simulink model "AmbTFilMgr"  (R2022b)
settings: Solver=FixedStepDiscrete  StartTime=0.0  StopTime=10.0  FixedStep=1  RelTol=1e-3
settings Model: Creator=blue.ge  LastModifiedBy=blue.ge
settings DataIOCC: SaveFormat=StructureWithTime
settings OptimizationCC: DefaultParameterBehavior=Inlined  EnhancedBackFolding=on  PassReuseOutputArgsAs=Individual arguments  ZeroExternalMemoryAtStartup=off  ZeroInternalMemoryAtStartup=off  OptimizeBlockOrder=speed  DifferentSizesBufferReuse=on  OptimizationCustomize=off  EfficientTunableParamExpr=on
settings DebuggingCC: ParameterTunabilityLossMsg=error
settings ModelReferenceCC: ModelReferenceNumInstancesAllowed=Single
settings SFSimCC: MATLABDynamicMemAlloc=off
settings RTWCC: Description=AUTOSAR  SystemTargetFile=autosar.tlc  GenCodeOnly=on  TemplateMakefile=ert_default_tmf
settings CodeAppCC: ForceParamTrailComments=on  IgnoreCustomStorageClasses=off  OperatorAnnotations=on  SimulinkDataObjDesc=on  SFDataObjDesc=on  InsertBlockDesc=on
settings STFCustomTargetCC: TargetLangStandard=C99 (ISO)  MultiwordLength=256  ParMdlRefBuildCompliant=on  CompOptLevelCompliant=on  IncludeMdlTerminateFcn=off  CombineOutputUpdateFcns=on  SuppressErrorStatus=on  ERTCustomFileBanners=on  SupportNonFinite=off  SupportComplex=off  SupportContinuousTime=off  SupportNonInlinedSFcns=off  RemoveResetFunc=on  MATLABClassNameForMDSCustomization=Simulink.SoftwareTarget.ERTCustomization  AutosarCompliant=on  UseToolchainInfoCompliant=on  LookupTableObjectStructAxisOrder=2,1,3,4,...  LUTObjectStructOrderEvenSpacing=Size,Table,Breakpoints  SystemTargetFile=autosar.tlc
settings ERTTargetCC: TargetLangStandard=C99 (ISO)  ConcurrentExecutionCompliant=off  IncludeMdlTerminateFcn=off  SuppressErrorStatus=on  SupportNonFinite=off  SupportComplex=off  ModelStepFunctionPrototypeControlCompliant=off  CPPClassGenCompliant=off  AutosarCompliant=on  LookupTableObjectStructAxisOrder=2,1,3,4,...  LUTObjectStructOrderEvenSpacing=Size,Table,Breakpoints  InlinedParameterPlacement=NonHierarchical  ERTCustomFileTemplate=example_file_process.tlc

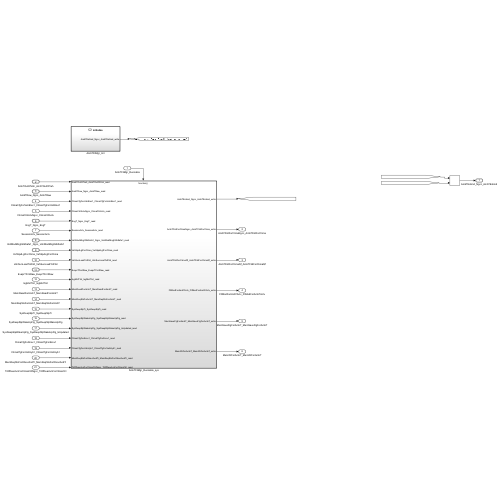
[thumbnail: Simulink's stored preview of the top-level diagram]
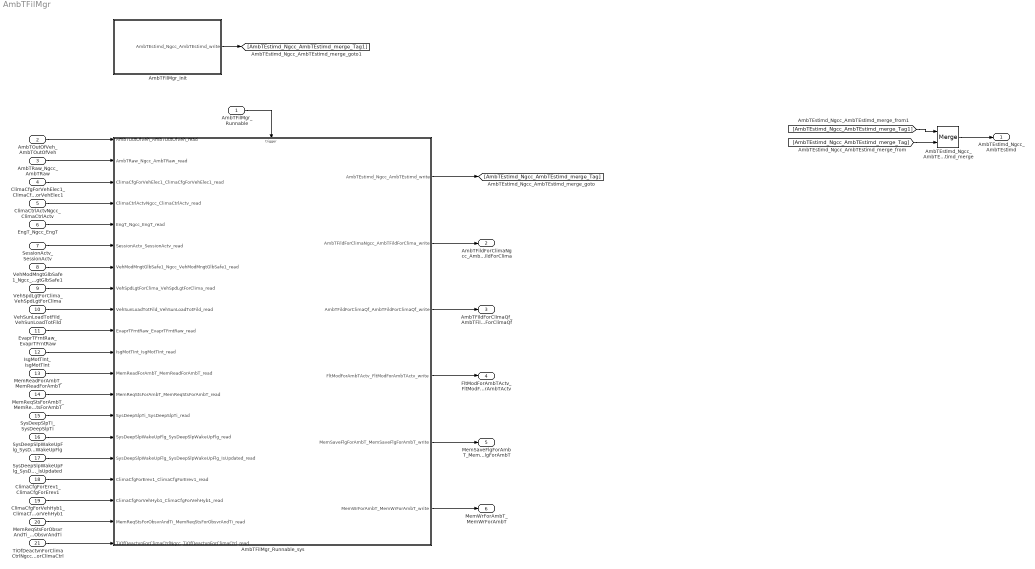
== system AmbTFilMgr ==
  AmbTFilMgr_Runnable : Inport  SampleTime=1  OutputFunctionCall=on
  AmbTOutOfVeh_AmbTOutOfVeh : Inport  Port=2  OutDataTypeStr=Bus: AmbTOutOfVeh  PortDimensions=1  BusOutputAsStruct=on
  AmbTRaw_Ngcc_AmbTRaw : Inport  Port=3  OutDataTypeStr=Bus: AmbTWithQly  PortDimensions=1  BusOutputAsStruct=on
  ClimaCfgForVehElec1_ClimaCfgForVehElec1 : Inport  Port=4  OutDataTypeStr=Boolean  PortDimensions=1
  ClimaCtrlActvNgcc_ClimaCtrlActv : Inport  Port=5  OutDataTypeStr=Boolean  PortDimensions=1
  EngT_Ngcc_EngT : Inport  Port=6  OutDataTypeStr=Bus: EngT  PortDimensions=1  BusOutputAsStruct=on
  SessionActv_SessionActv : Inport  Port=7  OutDataTypeStr=Enum: NoYes1  PortDimensions=1
  VehModMngtGlbSafe1_Ngcc_VehModMngtGlbSafe1 : Inport  Port=8  OutDataTypeStr=Bus: VehModMngtGlbSafe1  PortDimensions=1  BusOutputAsStruct=on
  VehSpdLgtForClima_VehSpdLgtForClima : Inport  Port=9  OutDataTypeStr=Bus: VehSpdLgtForClima  PortDimensions=1  BusOutputAsStruct=on
  VehSunLoadTotFild_VehSunLoadTotFild : Inport  Port=10  OutDataTypeStr=SunLoad30  PortDimensions=1
  EvaprTFrntRaw_EvaprTFrntRaw : Inport  Port=11  OutDataTypeStr=Bus: EvaprTFrnt  PortDimensions=1  BusOutputAsStruct=on
  IsgMotTInt_IsgMotTInt : Inport  Port=12  OutDataTypeStr=T3  PortDimensions=1
  MemReadForAmbT_MemReadForAmbT : Inport  Port=13  OutDataTypeStr=T30  PortDimensions=1
  MemReqStsForAmbT_MemReqStsForAmbT : Inport  Port=14  OutDataTypeStr=Enum: MemReqSts  PortDimensions=1
  SysDeepSlpTi_SysDeepSlpTi : Inport  Port=15  OutDataTypeStr=CarTiGlb  PortDimensions=1
  SysDeepSlpWakeUpFlg_SysDeepSlpWakeUpFlg : Inport  Port=16  OutDataTypeStr=Boolean  PortDimensions=1
  SysDeepSlpWakeUpFlg_SysDeepSlpWakeUpFlg_IsUpdated : Inport  Port=17  OutDataTypeStr=boolean  PortDimensions=1
  ClimaCfgForErev1_ClimaCfgForErev1 : Inport  Port=18  OutDataTypeStr=Boolean  PortDimensions=1
  ClimaCfgForVehHyb1_ClimaCfgForVehHyb1 : Inport  Port=19  OutDataTypeStr=Boolean  PortDimensions=1
  MemReqStsForObsvrAndTi_MemReqStsForObsvrAndTi : Inport  Port=20  OutDataTypeStr=Enum: MemReqSts  PortDimensions=1
  TiOfDeactvnForClimaCtrlNgcc_TiOfDeactvnForClimaCtrl : Inport  Port=21  OutDataTypeStr=Ti33  PortDimensions=1
  AmbTEstimd_Ngcc_AmbTEstimd_merge : Merge
  AmbTFilMgr_Init : Subsystem
  AmbTFilMgr_Runnable_sys : Subsystem
  AmbTEstimd_Ngcc_AmbTEstimd : Outport  OutDataTypeStr=Bus: AmbTEstimd  PortDimensions=1  BusOutputAsStruct=on
  AmbTFildForClimaNgcc_AmbTFildForClima : Outport  Port=2  OutDataTypeStr=T30  PortDimensions=1
  AmbTFildForClimaQf_AmbTFildForClimaQf : Outport  Port=3  OutDataTypeStr=Enum: GenQf1  PortDimensions=1
  FltModForAmbTActv_FltModForAmbTActv : Outport  Port=4  OutDataTypeStr=Boolean  PortDimensions=1
  MemSaveFlgForAmbT_MemSaveFlgForAmbT : Outport  Port=5  OutDataTypeStr=Boolean  PortDimensions=1
  MemWrForAmbT_MemWrForAmbT : Outport  Port=6  OutDataTypeStr=T30  PortDimensions=1
  wires:
  AmbTOutOfVeh_AmbTOutOfVeh/1 -> AmbTFilMgr_Runnable_sys/1(AmbTOutOfVeh_AmbTOutOfVeh_read)
  AmbTRaw_Ngcc_AmbTRaw/1 -> AmbTFilMgr_Runnable_sys/2(AmbTRaw_Ngcc_AmbTRaw_read)
  ClimaCfgForVehElec1_ClimaCfgForVehElec1/1 -> AmbTFilMgr_Runnable_sys/3(ClimaCfgForVehElec1_ClimaCfgForVehElec1_read)
  ClimaCtrlActvNgcc_ClimaCtrlActv/1 -> AmbTFilMgr_Runnable_sys/4(ClimaCtrlActvNgcc_ClimaCtrlActv_read)
  EngT_Ngcc_EngT/1 -> AmbTFilMgr_Runnable_sys/5(EngT_Ngcc_EngT_read)
  SessionActv_SessionActv/1 -> AmbTFilMgr_Runnable_sys/6(SessionActv_SessionActv_read)
  VehModMngtGlbSafe1_Ngcc_VehModMngtGlbSafe1/1 -> AmbTFilMgr_Runnable_sys/7(VehModMngtGlbSafe1_Ngcc_VehModMngtGlbSafe1_read)
  VehSpdLgtForClima_VehSpdLgtForClima/1 -> AmbTFilMgr_Runnable_sys/8(VehSpdLgtForClima_VehSpdLgtForClima_read)
  VehSunLoadTotFild_VehSunLoadTotFild/1 -> AmbTFilMgr_Runnable_sys/9(VehSunLoadTotFild_VehSunLoadTotFild_read)
  EvaprTFrntRaw_EvaprTFrntRaw/1 -> AmbTFilMgr_Runnable_sys/10(EvaprTFrntRaw_EvaprTFrntRaw_read)
  IsgMotTInt_IsgMotTInt/1 -> AmbTFilMgr_Runnable_sys/11(IsgMotTInt_IsgMotTInt_read)
  MemReadForAmbT_MemReadForAmbT/1 -> AmbTFilMgr_Runnable_sys/12(MemReadForAmbT_MemReadForAmbT_read)
  MemReqStsForAmbT_MemReqStsForAmbT/1 -> AmbTFilMgr_Runnable_sys/13(MemReqStsForAmbT_MemReqStsForAmbT_read)
  SysDeepSlpTi_SysDeepSlpTi/1 -> AmbTFilMgr_Runnable_sys/14(SysDeepSlpTi_SysDeepSlpTi_read)
  SysDeepSlpWakeUpFlg_SysDeepSlpWakeUpFlg/1 -> AmbTFilMgr_Runnable_sys/15(SysDeepSlpWakeUpFlg_SysDeepSlpWakeUpFlg_read)
  SysDeepSlpWakeUpFlg_SysDeepSlpWakeUpFlg_IsUpdated/1 -> AmbTFilMgr_Runnable_sys/16(SysDeepSlpWakeUpFlg_SysDeepSlpWakeUpFlg_IsUpdated_read)
  ClimaCfgForErev1_ClimaCfgForErev1/1 -> AmbTFilMgr_Runnable_sys/17(ClimaCfgForErev1_ClimaCfgForErev1_read)
  ClimaCfgForVehHyb1_ClimaCfgForVehHyb1/1 -> AmbTFilMgr_Runnable_sys/18(ClimaCfgForVehHyb1_ClimaCfgForVehHyb1_read)
  MemReqStsForObsvrAndTi_MemReqStsForObsvrAndTi/1 -> AmbTFilMgr_Runnable_sys/19(MemReqStsForObsvrAndTi_MemReqStsForObsvrAndTi_read)
  TiOfDeactvnForClimaCtrlNgcc_TiOfDeactvnForClimaCtrl/1 -> AmbTFilMgr_Runnable_sys/20(TiOfDeactvnForClimaCtrlNgcc_TiOfDeactvnForClimaCtrl_read)
  AmbTEstimd_Ngcc_AmbTEstimd_merge/1 -> AmbTEstimd_Ngcc_AmbTEstimd/1
  AmbTFilMgr_Runnable_sys/2(AmbTFildForClimaNgcc_AmbTFildForClima_write) -> AmbTFildForClimaNgcc_AmbTFildForClima/1
  AmbTFilMgr_Runnable_sys/3(AmbTFildForClimaQf_AmbTFildForClimaQf_write) -> AmbTFildForClimaQf_AmbTFildForClimaQf/1
  AmbTFilMgr_Runnable_sys/4(FltModForAmbTActv_FltModForAmbTActv_write) -> FltModForAmbTActv_FltModForAmbTActv/1
  AmbTFilMgr_Runnable_sys/5(MemSaveFlgForAmbT_MemSaveFlgForAmbT_write) -> MemSaveFlgForAmbT_MemSaveFlgForAmbT/1
  AmbTFilMgr_Runnable_sys/6(MemWrForAmbT_MemWrForAmbT_write) -> MemWrForAmbT_MemWrForAmbT/1
  AmbTFilMgr_Runnable_sys/1(AmbTEstimd_Ngcc_AmbTEstimd_write) -> [AmbTEstimd_Ngcc_AmbTEstimd_merge_Tag]
  [AmbTEstimd_Ngcc_AmbTEstimd_merge_Tag] -> AmbTEstimd_Ngcc_AmbTEstimd_merge/2
  AmbTFilMgr_Init/1(AmbTEstimd_Ngcc_AmbTEstimd_write) -> [AmbTEstimd_Ngcc_AmbTEstimd_merge_Tag1]
  [AmbTEstimd_Ngcc_AmbTEstimd_merge_Tag1] -> AmbTEstimd_Ngcc_AmbTEstimd_merge/1
  AmbTFilMgr_Runnable/1 -> AmbTFilMgr_Runnable_sys/Trigger

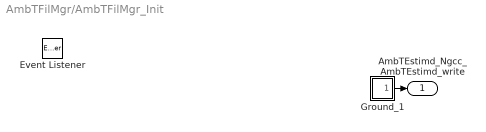
== system AmbTFilMgr/AmbTFilMgr_Init ==
  Event Listener : EventListener  EventName=reset
  Ground_1 : Subsystem
  AmbTEstimd_Ngcc_AmbTEstimd_write : Outport
  wires:
  Ground_1/1(Out1) -> AmbTEstimd_Ngcc_AmbTEstimd_write/1

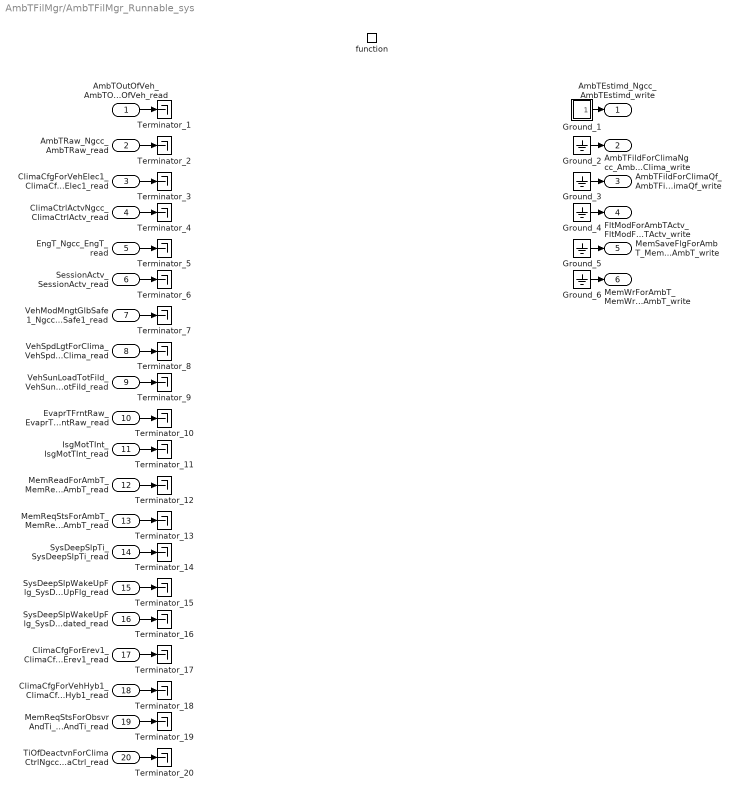
== system AmbTFilMgr/AmbTFilMgr_Runnable_sys ==
  AmbTOutOfVeh_AmbTOutOfVeh_read : Inport
  AmbTRaw_Ngcc_AmbTRaw_read : Inport  Port=2
  ClimaCfgForVehElec1_ClimaCfgForVehElec1_read : Inport  Port=3
  ClimaCtrlActvNgcc_ClimaCtrlActv_read : Inport  Port=4
  EngT_Ngcc_EngT_read : Inport  Port=5
  SessionActv_SessionActv_read : Inport  Port=6
  VehModMngtGlbSafe1_Ngcc_VehModMngtGlbSafe1_read : Inport  Port=7
  VehSpdLgtForClima_VehSpdLgtForClima_read : Inport  Port=8
  VehSunLoadTotFild_VehSunLoadTotFild_read : Inport  Port=9
  EvaprTFrntRaw_EvaprTFrntRaw_read : Inport  Port=10
  IsgMotTInt_IsgMotTInt_read : Inport  Port=11
  MemReadForAmbT_MemReadForAmbT_read : Inport  Port=12
  MemReqStsForAmbT_MemReqStsForAmbT_read : Inport  Port=13
  SysDeepSlpTi_SysDeepSlpTi_read : Inport  Port=14
  SysDeepSlpWakeUpFlg_SysDeepSlpWakeUpFlg_read : Inport  Port=15
  SysDeepSlpWakeUpFlg_SysDeepSlpWakeUpFlg_IsUpdated_read : Inport  Port=16
  ClimaCfgForErev1_ClimaCfgForErev1_read : Inport  Port=17
  ClimaCfgForVehHyb1_ClimaCfgForVehHyb1_read : Inport  Port=18
  MemReqStsForObsvrAndTi_MemReqStsForObsvrAndTi_read : Inport  Port=19
  TiOfDeactvnForClimaCtrlNgcc_TiOfDeactvnForClimaCtrl_read : Inport  Port=20
  function : TriggerPort  TriggerType=function-call  VariantControl=(inherit)  SampleTimeType=periodic  SampleTime=-1
  Ground_1 : Subsystem
  Ground_{2..6} (5 alike) : Ground
  Terminator_{1..20} (20 alike) : Terminator
  AmbTEstimd_Ngcc_AmbTEstimd_write : Outport
      settings: EnsureOutportIsVirtual=on
  AmbTFildForClimaNgcc_AmbTFildForClima_write : Outport  Port=2
      settings: EnsureOutportIsVirtual=on
  AmbTFildForClimaQf_AmbTFildForClimaQf_write : Outport  Port=3
      settings: EnsureOutportIsVirtual=on
  FltModForAmbTActv_FltModForAmbTActv_write : Outport  Port=4
      settings: EnsureOutportIsVirtual=on
  MemSaveFlgForAmbT_MemSaveFlgForAmbT_write : Outport  Port=5
      settings: EnsureOutportIsVirtual=on
  MemWrForAmbT_MemWrForAmbT_write : Outport  Port=6
      settings: EnsureOutportIsVirtual=on
  wires:
  AmbTOutOfVeh_AmbTOutOfVeh_read/1 -> Terminator_1/1
  AmbTRaw_Ngcc_AmbTRaw_read/1 -> Terminator_2/1
  ClimaCfgForVehElec1_ClimaCfgForVehElec1_read/1 -> Terminator_3/1
  ClimaCtrlActvNgcc_ClimaCtrlActv_read/1 -> Terminator_4/1
  EngT_Ngcc_EngT_read/1 -> Terminator_5/1
  SessionActv_SessionActv_read/1 -> Terminator_6/1
  VehModMngtGlbSafe1_Ngcc_VehModMngtGlbSafe1_read/1 -> Terminator_7/1
  VehSpdLgtForClima_VehSpdLgtForClima_read/1 -> Terminator_8/1
  VehSunLoadTotFild_VehSunLoadTotFild_read/1 -> Terminator_9/1
  EvaprTFrntRaw_EvaprTFrntRaw_read/1 -> Terminator_10/1
  IsgMotTInt_IsgMotTInt_read/1 -> Terminator_11/1
  MemReadForAmbT_MemReadForAmbT_read/1 -> Terminator_12/1
  MemReqStsForAmbT_MemReqStsForAmbT_read/1 -> Terminator_13/1
  SysDeepSlpTi_SysDeepSlpTi_read/1 -> Terminator_14/1
  SysDeepSlpWakeUpFlg_SysDeepSlpWakeUpFlg_read/1 -> Terminator_15/1
  SysDeepSlpWakeUpFlg_SysDeepSlpWakeUpFlg_IsUpdated_read/1 -> Terminator_16/1
  ClimaCfgForErev1_ClimaCfgForErev1_read/1 -> Terminator_17/1
  ClimaCfgForVehHyb1_ClimaCfgForVehHyb1_read/1 -> Terminator_18/1
  MemReqStsForObsvrAndTi_MemReqStsForObsvrAndTi_read/1 -> Terminator_19/1
  TiOfDeactvnForClimaCtrlNgcc_TiOfDeactvnForClimaCtrl_read/1 -> Terminator_20/1
  Ground_1/1(Out1) -> AmbTEstimd_Ngcc_AmbTEstimd_write/1
  Ground_2/1 -> AmbTFildForClimaNgcc_AmbTFildForClima_write/1
  Ground_3/1 -> AmbTFildForClimaQf_AmbTFildForClimaQf_write/1
  Ground_4/1 -> FltModForAmbTActv_FltModForAmbTActv_write/1
  Ground_5/1 -> MemSaveFlgForAmbT_MemSaveFlgForAmbT_write/1
  Ground_6/1 -> MemWrForAmbT_MemWrForAmbT_write/1

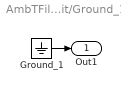
== system AmbTFilMgr/AmbTFilMgr_Init/Ground_1 ==
  Ground_1 : Ground
  Out1 : Outport  OutDataTypeStr=Bus: AmbTEstimd  PortDimensions=1
  wires:
  Ground_1/1 -> Out1/1

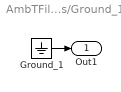
== system AmbTFilMgr/AmbTFilMgr_Runnable_sys/Ground_1 ==
  Ground_1 : Ground
  Out1 : Outport  OutDataTypeStr=Bus: AmbTEstimd  PortDimensions=1
  wires:
  Ground_1/1 -> Out1/1
